simulink model "HDVSim_BEV_WPT_Truck"  (R2018b) (continued)
== system HDVSim_BEV_WPT_Truck/Electric == (continued)
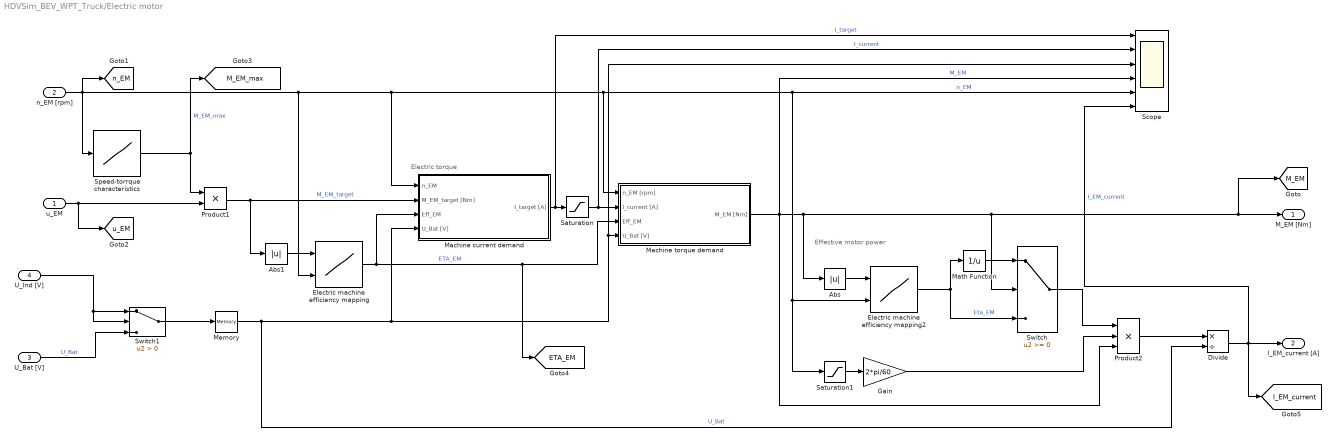
== system HDVSim_BEV_WPT_Truck/Electric motor ==
  note: Electric torque
  note: Effective motor power
  u_EM : Inport
  n_EM [rpm] : Inport  Port=2
  U_Bat [V] : Inport  Port=3
  U_Ind [V] : Inport  Port=4
  Abs : Abs
  Abs1 : Abs
  Divide : Product  Inputs=*/
  Electric machine efficiency mapping : Lookup_n-D  BreakpointsForDimension1=em.efficiency.torque  BreakpointsForDimension2=em.efficiency.speed  Table=em.efficiency.characteristic_map  RndMeth=Simplest  InputSameDT=on
  Electric machine efficiency mapping2 : Lookup_n-D  BreakpointsForDimension1=em.efficiency.torque  BreakpointsForDimension2=em. efficiency.speed  Table=em.efficiency.characteristic_map  RndMeth=Simplest  InputSameDT=on
  Gain : Gain  Gain=2*pi/60
  Machine current demand : Subsystem
  Machine torque demand : Subsystem
  Math Function : Math  Operator=reciprocal  SaturateOnIntegerOverflow=on
  Memory : Memory  InitialCondition=1.0943*Bat.Voltage
  Product1 : Product
  Product2 : Product  Inputs=3
  Saturation : Saturate  UpperLimit=600  LowerLimit=-600
  Saturation1 : Saturate  UpperLimit=inf  LowerLimit=1
  Scope : Scope  NumInputPorts=6
  Speed-torrque characteristics : Lookup_n-D  NumberOfTableDimensions=1  BreakpointsForDimension1=em.speed  Table=em.trq  ExtrapMethod=Clip  RndMeth=Simplest  InputSameDT=on
  Switch : Switch
  Switch1 : Switch  Criteria=u2 > Threshold
  M_EM [Nm] : Outport
  I_EM_current [A] : Outport  Port=2
  tag scope: ETA_EM=global, I_EM_current=global, M_EM=global, M_EM_max=global, n_EM=global, u_EM=global
  wires:
  Speed-torrque characteristics/1 -> [M_EM_max], Product1/1  "M_EM_max"
  u_EM/1 -> Product1/2, [u_EM]
  U_Bat [V]/1 -> Switch1/3  "U_Bat"
  Machine current demand/1(I_target [A]) -> Scope/1, Saturation/1  "I_target"
  Saturation/1 -> Scope/2, Machine torque demand/2(I_current [A])  "I_current"
  Machine torque demand/1(M_EM [Nm]) -> Scope/4, Product2/3, [M_EM], M_EM [Nm]/1, Switch/2, Abs/1  "M_EM"
  Gain/1 -> Product2/2
  Divide/1 -> [I_EM_current], Scope/6, I_EM_current [A]/1  "I_EM_current"
  Math Function/1 -> Switch/1
  Electric machine efficiency mapping2/1 -> Switch/3, Math Function/1  "Eta_EM"
  Switch/1 -> Product2/1
  Product2/1 -> Divide/1
  Abs/1 -> Electric machine efficiency mapping2/1
  Product1/1 -> Machine current demand/2(M_EM_target [Nm]), Abs1/1  "M_EM_target"
  n_EM [rpm]/1 -> Speed-torrque characteristics/1, Electric machine efficiency mapping/2, Machine current demand/1("n_EM "), Machine torque demand/1(n_EM [rpm]), Saturation1/1, Electric machine efficiency mapping2/2, Scope/5, [n_EM]  "n_EM"
  Abs1/1 -> Electric machine efficiency mapping/1
  Electric machine efficiency mapping/1 -> Machine current demand/3(Eff_EM), [ETA_EM], Machine torque demand/3(Eff_EM)  "ETA_EM"
  Saturation1/1 -> Gain/1
  Memory/1 -> Divide/2, Scope/3, Machine torque demand/4(U_Bat [V]), Machine current demand/4(U_Bat [V])  "U_Bat"
  Switch1/1 -> Memory/1
  U_Ind [V]/1 -> Switch1/2, Switch1/1

== system HDVSim_BEV_WPT_Truck/Hybrid ==
  Abs : Abs
  Abs1 : Abs
  Constant1 : Constant  Value=0
  Display1 : Display
  Display5 : Display
  Display6 : Display
  Gain1 : Gain  Gain=1/3600000
  Gain6 : Gain  Gain=1/3600000
  Gain7 : Gain  Gain=1/3600000
  Integrator1 : Integrator
  Integrator5 : Integrator
  Integrator6 : Integrator
  Out_Bat : Scope  NumInputPorts=4
  Product1 : Product
  Product3 : Product
  Scope1 : Scope
  Scope3 : Scope
  Scope7 : Scope  NumInputPorts=2
  Switch1 : Switch  Threshold=1
  wires:
  Product1/1 -> Switch1/1, Integrator6/1
  Gain1/1 -> Scope7/1, Display1/1  "E_REKUP_ES_AN [kWh]"
  Constant1/1 -> Switch1/3
  Integrator1/1 -> Gain1/1
  Switch1/1 -> Integrator1/1
  [REKUP] -> Scope7/2, Switch1/2  "REKUP"
  [U_Bat] -> Product1/2
  [I_current_BAT] -> Product1/1
  [SOC] -> Out_Bat/1
  Gain7/1 -> Display6/1, Scope1/1
  Integrator6/1 -> Gain7/1
  [U_Bat] -> Abs1/1
  [I_current_BAT] -> Abs/1
  Product3/1 -> Integrator5/1
  Gain6/1 -> Display5/1, Scope3/1
  Integrator5/1 -> Gain6/1
  Abs/1 -> Product3/1
  Abs1/1 -> Product3/2
  [s_current1] -> Out_Bat/2
  [C_Rate] -> Out_Bat/3
  [I_EM_current] -> Out_Bat/4

== system HDVSim_BEV_WPT_Truck/Inductive charging ==
  v_target [m/s] : Inport
  Compare To Constant1 : simulink/Logic and Bit Operations/Compare To Constant  relop=<=  const=WPT.expansion  OutDataTypeStr=boolean
  Compare To Constant2 : simulink/Logic and Bit Operations/Compare To Constant  relop=>=  const=78  OutDataTypeStr=boolean
  Compare To Constant3 : simulink/Logic and Bit Operations/Compare To Constant  relop=>  const=0  OutDataTypeStr=boolean
  Constant : Constant  Value=WPT.Voltage
  Constant1 : Constant  Value=0
  Gain : Gain  Gain=3.6
  Logical Operator : Logic  AllPortsSameDT=off  OutDataTypeStr=boolean
  Rate Transition : RateTransition
  Scope : Scope
  Scope1 : Scope  NumInputPorts=2
  Switch : Switch  Criteria=u2 ~= 0  Threshold=78
  Terminator : Terminator
  Uniform Random Number1 : UniformRandomNumber  Minimum=0  SampleTime=10
  U_Ind : Outport
  wires:
  v_target [m/s]/1 -> Gain/1
  Gain/1 -> Compare To Constant2/1
  Constant/1 -> Switch/1
  Constant1/1 -> Switch/3
  Switch/1 -> Scope/1, U_Ind/1
  Uniform Random Number1/1 -> Rate Transition/1
  Compare To Constant1/1 -> Logical Operator/1, Scope1/2
  Logical Operator/1 -> Switch/2
  Compare To Constant2/1 -> Logical Operator/2
  Rate Transition/1 -> Scope1/1, Compare To Constant1/1
  [slope_q] -> Compare To Constant3/1
  Compare To Constant3/1 -> Terminator/1

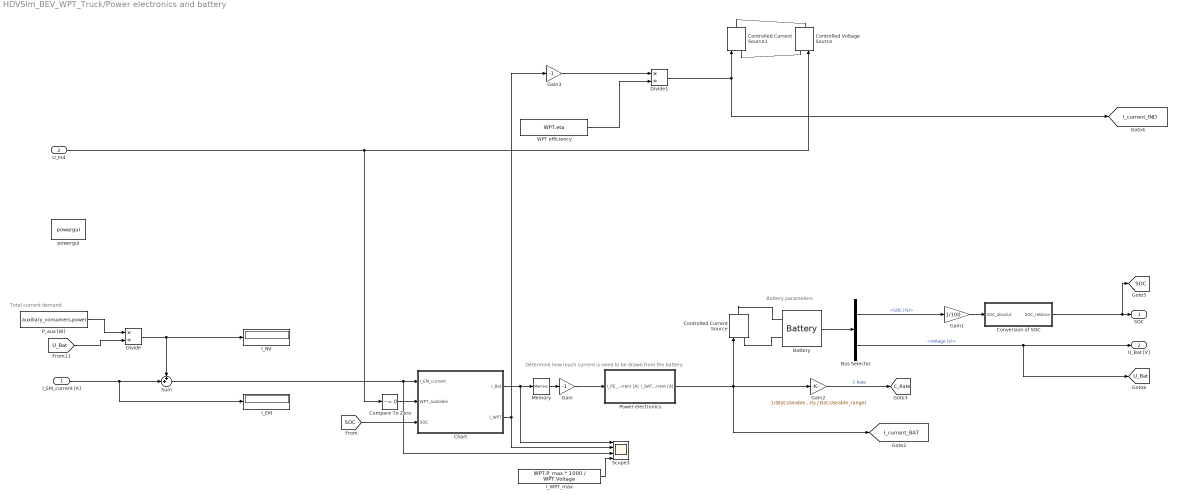
== system HDVSim_BEV_WPT_Truck/Power electronics and battery ==
  note: Total current demand
  note: Battery parameters
  note: Determine how much current is need to be drawn from the battery
  I_EM_current [A] : Inport
  U_Ind : Inport  Port=2
  Battery : electricdrivelib/Extra Sources/Battery  BatType=Lithium-Ion  ShowTempParam=off  ThermalPreset=no  ShowAgeParam=off  NomV=Bat.Voltage  NomQ=Bat.Useable_capacity / Bat.Useable_range  SOC=(Bat.SOC_start *Bat.Useable_range+(0.5-Bat.Useable_range/2))* 100  Batt_Tr=30  PresetModel=off  MaxQ=Bat.Useable_capacity / Bat.Useable_range *8077/7500  MinV=487.5  FullV=Bat.Voltage * 1.178  Dis_rate=0.2 * Bat.Useable_capacity / Bat.Useable_range  R=0.01 * Bat.Voltage / (Bat.Useable_capacity / Bat.Useable_range)  Normal_OP=Bat.Useable_capacity / Bat.Useable_range * 0.962  expZone="[Bat.Voltage*4339/4000     Bat.Useable_capacity / Bat.Useable_range/5]"  current=[5 25 50 250]  Units=Ampere-hour  Tci=20  NomT=20  T2=-30  MaxQ2=4.8  FullV2=7.1  Normal_OP2=5.72  expZone2="[6.58  1]"  Rca=0.6  ti=1000  Dp=0  Neq0=0  Tsim=1e6  Ta1=25  Qeol=5.4*0.9  Reol=0.013333*1.2  Char_age=[2.3478, 3]  Dis_age=[2.3478, 10]  N1=1500  N2=10500  N3=1000  N4=1400  Ta2=45  N5=950
      settings: SourceProductName=Simscape Electrical
  Bus Selector : BusSelector  OutputSignals=SOC (%),Voltage (V)
  Chart : Stateflow chart  TreatAsAtomicUnit=on
  Compare To Zero : simulink/Logic and Bit Operations/Compare To Zero  relop=~=  OutDataTypeStr=boolean
  Controlled Current Source : powerlib/Electrical Sources/Controlled Current Source  Initialize=off  Source_Type=DC  Amplitude=0  Phase=0  Frequency=0  Measurements=None
      settings: SourceProductName=Simscape Electrical
  Controlled Current Source1 : powerlib/Electrical Sources/Controlled Current Source  Initialize=off  Source_Type=DC  Amplitude=0  Phase=0  Frequency=0  Measurements=None
      settings: SourceProductName=Simscape Electrical
  Controlled Voltage Source : powerlib/Electrical Sources/Controlled Voltage Source  Initialize=on  Source_Type=DC  Amplitude=0  Phase=0  Frequency=0  Measurements=None
      settings: SourceProductName=Simscape Electrical
  Conversion of SOC : Subsystem
  Divide : Product  Inputs=*/
  Divide1 : Product  Inputs=*/
  Gain : Gain  Gain=-1
  Gain1 : Gain  Gain=1/100
  Gain2 : Gain  Gain=1/(Bat.Useable_capacity / Bat.Useable_range)
  Gain3 : Gain  Gain=-1
  I_EM : Display
  I_NV : Display
  I_WPT_max : Constant  Value=WPT.P_max * 1000 / WPT.Voltage
  Memory : Memory
  P_aux [W] : Constant  Value=auxiliary_consumers.power
  Power electronics : Subsystem
  Scope3 : Scope  NumInputPorts=4
  Sum : Sum  Inputs=++|
  WPT efficiency : Constant  Value=WPT.eta
  powergui : powerlib/powergui  Priority=1  SimulationMode=Discrete  SampleTime=sample_time  frequency=60  Iterations=50  frequencyindice=0  Pbase=100e6  ErrMax=1e-4  UnitsV=kV  UnitsW=MW  buscounter=0  FunctionMessages=off  echomessages=off  EnableUseOfTLC=off  CurrentSourceSwitches=off  DisableSnubberDevices=off  DisableRonSwitches=off  DisableVfSwitches=off  DisplayEquations=off  SolverType=Tustin  Interpol=off  ExternalGateDelay=off  methode=off  Ts=100  x0status=blocks  SPID=off  SwTol=0  HookPort=off  RestoreLinks=warning  ResistiveCurrentMeasurement=off  Frange=[0:2:500]  Ylog=off  Xlog=on  ShowGrid=off  save=off  variable=ZData  structure=0  ZoomFFT=off  StartTime=0.0  cycles=1  DisplayStyle=1  fundamental=60  FreqAxis=off  MaxFrequency=1000  frequencyindicesteady=1  RmsSteady=1  display=off
      settings: SourceProductName=Simscape Electrical
  SOC : Outport
  U_Bat [V] : Outport  Port=2
  tag scope: C_Rate=global, I_current_BAT=global, I_current_IND=global, SOC=global, U_Bat=global
  wires:
  Battery/1 -> Bus Selector/1
  Bus Selector/1 -> Gain1/1  "<SOC (%)>"
  Bus Selector/2 -> [U_Bat], U_Bat [V]/1  "<Voltage (V)>"
  Gain1/1 -> Conversion of SOC/1(SOC_absolut)
  Conversion of SOC/1(SOC_relative) -> [SOC], SOC/1
  Memory/1 -> Gain/1
  Gain/1 -> Power electronics/1(I_PE_current [A])
  Gain2/1 -> [C_Rate]  "C Rate"
  [U_Bat] -> Divide/2
  P_aux [W]/1 -> Divide/1
  Divide/1 -> Sum/1, I_NV/1
  Sum/1 -> Scope3/3, Chart/1(I_EM_current)
  U_Ind/1 -> Controlled Voltage Source/1, Compare To Zero/1
  Chart/1(I_Bat) -> Memory/1, Scope3/1
  Chart/2(I_WPT) -> Scope3/2, Gain3/1
  Compare To Zero/1 -> Chart/2(WPT_available)
  Gain3/1 -> Divide1/1
  Divide1/1 -> [I_current_IND], Controlled Current Source1/1
  WPT efficiency/1 -> Divide1/2
  I_WPT_max/1 -> Scope3/4
  Power electronics/1(I_BAT_current [A]) -> [I_current_BAT], Gain2/1, Controlled Current Source/1
  [SOC] -> Chart/3(SOC)
  I_EM_current [A]/1 -> I_EM/1, Sum/2
  connections:
  Controlled Current Source/LConn1 -- Battery/LConn2
  Controlled Current Source/RConn1 -- Battery/LConn1
  Controlled Current Source1/RConn1 -- Controlled Voltage Source/RConn1
  Controlled Current Source1/LConn1 -- Controlled Voltage Source/LConn1

== system HDVSim_BEV_WPT_Truck/Tires ==
  M_tire [Nm] : Inport
  v_current [m/s] : Inport  Port=2
  F=M/r : Product  Inputs=*/
  "phi. -> n[1/min]" : Gain  Gain=60/2/pi
  phi. = v/r : Product  Inputs=/*
  tire radius : Constant  Value=tires.radius
  n_tire [rpm] : Outport
  F_x [N] : Outport  Port=2
  wires:
  phi. = v/r/1 -> "phi. -> n[1/min]"/1
  "phi. -> n[1/min]"/1 -> n_tire [rpm]/1
  tire radius/1 -> phi. = v/r/1, F=M/r/2
  v_current [m/s]/1 -> phi. = v/r/2
  M_tire [Nm]/1 -> F=M/r/1
  F=M/r/1 -> F_x [N]/1

== system HDVSim_BEV_WPT_Truck/Transmission ==
  note: Transmission torque multiplication through the different gears
  M_EM [Nm] : Inport
  n_Gearbox [rpm] : Inport  Port=2
  delta_v : Inport  Port=3
  v_target [m/s] : Inport  Port=4
  alpha [rad] : Inport  Port=5
  Determine when shifting occurs : Subsystem  TreatAsAtomicUnit=on
  Memory1 : Memory
  Number of gears : Constant  Value=length(transmission.ratios)
  Saturation : Saturate  UpperLimit=inf  LowerLimit=0
  Shifting rules : Stateflow chart  TreatAsAtomicUnit=on
  Torque flow through the transmission : Subsystem
  ZKU : Subsystem
  init_gear : Constant  Value=transmission.init_gear
  n_EM [rpm] : Outport
  M_Gearbox [Nm] : Outport  Port=2
  tag scope: gear=global, i_gearbox=global, recommend_DOWN_now=global, recommend_UP_now=global
  wires:
  n_Gearbox [rpm]/1 -> Torque flow through the transmission/2(n_gearbox_out [1/min]), Determine when shifting occurs/2(n_Getr_out [1/min])
  Torque flow through the transmission/2(M_gearbox_out [Nm]) -> [M_Getr_out], M_Gearbox [Nm]/1
  Torque flow through the transmission/1(n_gearbox_in [1/min]) -> Saturation/1
  init_gear/1 -> Shifting rules/1(init_gear)
  Determine when shifting occurs/27(check_torque_reseve_2lower) -> Shifting rules/29(check_torque_reserve_2lower)
  Determine when shifting occurs/26(check_torque_reseve_2higher) -> Shifting rules/28(check_torque_reserve_2higher)
  Determine when shifting occurs/1(reccommend_down_2higher) -> Shifting rules/3(recommend_down_2higher)
  Determine when shifting occurs/2(reccommend_down_1higher) -> Shifting rules/4(recommend_down_1higher)
  Determine when shifting occurs/3(reccommend_down_now) -> Shifting rules/5(recommend_down_now), [recommend_DOWN_now]
  Determine when shifting occurs/4(reccommend_down_1lower) -> Shifting rules/6(recommend_down_1lower)
  Determine when shifting occurs/5(reccommend_down_2lower) -> Shifting rules/7(recommend_down_2llower)
  Determine when shifting occurs/6(reccommend_up_2higher) -> Shifting rules/8(recommend_up_2higher)
  Determine when shifting occurs/7(reccommend_up_1higher) -> Shifting rules/9(recommend_up_1higher)
  Determine when shifting occurs/8(reccommend_up_now) -> Shifting rules/10(recommend_up_now), [recommend_UP_now]
  Determine when shifting occurs/9(reccommend_up_1lower) -> Shifting rules/11(recommend_up_1lower)
  Determine when shifting occurs/10(reccommend_up_2lower) -> Shifting rules/12(recommend_up_2lower)
  Determine when shifting occurs/11(check_n_min_2higher) -> Shifting rules/13(check_n_min_2higher)
  Determine when shifting occurs/12(check_n_min_1higher) -> Shifting rules/14(check_n_min_1higher)
  Determine when shifting occurs/13(check_n_min_now) -> Shifting rules/15(check_n_min_now)
  Determine when shifting occurs/14(check_n_min_1lower) -> Shifting rules/16(check_n_min_1lower)
  Determine when shifting occurs/15(check_n_min_2lower) -> Shifting rules/17(check_n_min_2lower)
  Determine when shifting occurs/16(check_n_max_2higher) -> Shifting rules/18(check_n_max_2higher)
  Determine when shifting occurs/17(check_n_max_1higher) -> Shifting rules/19(check_n_max_1higher)
  Determine when shifting occurs/18(check_n_max_now) -> Shifting rules/20(check_n_max_now)
  Determine when shifting occurs/19(check_n_max_1lower) -> Shifting rules/21(check_n_max_1lower)
  Determine when shifting occurs/20(check_n_max_2lower) -> Shifting rules/22(check_n_max_2lower)
  Determine when shifting occurs/21(check_M_max_2higher) -> Shifting rules/23(check_M_max_2higher)  "check_M_max_2higher"
  Determine when shifting occurs/22(check_M_max_1higher) -> Shifting rules/24(check_M_max_1higher)  "check_M_max_1higher"
  Determine when shifting occurs/23(check_M_max_now) -> Shifting rules/25(check_M_max_now)  "check_M_max_now"
  Determine when shifting occurs/24(check_M_max_1lower) -> Shifting rules/26(check_M_max_1lower)  "check_M_max_1lower"
  Determine when shifting occurs/25(check_M_max_2lower) -> Shifting rules/27(check_M_max_2lower)  "check_M_max_2lower"
  Memory1/1 -> [gear], [Gear], ZKU/1(Gear), Torque flow through the transmission/3(Gear)
  M_EM [Nm]/1 -> Torque flow through the transmission/1(M_gearbox_in [Nm])
  Saturation/1 -> n_EM [rpm]/1
  Torque flow through the transmission/3(i_gearbox) -> [i_gearbox]
  delta_v/1 -> Determine when shifting occurs/4(delta_v)
  v_target [m/s]/1 -> Determine when shifting occurs/5(v_target [m/s])
  Number of gears/1 -> Shifting rules/2(z)
  alpha [rad]/1 -> Determine when shifting occurs/6(alpha [rad])
  Shifting rules/1(gear) -> [gear]
  [gear] -> Memory1/1
  [M_Getr_out] -> Determine when shifting occurs/3(M_Getr_out [Nm])
  [Gear] -> Determine when shifting occurs/1(Gear)

== system HDVSim_BEV_WPT_Truck/Vehicle ==
  note: Net tractive force applied on the vehicle
  note: Vehicle acceleration
  F_x [N] : Inport
  u_KB : Inport  Port=2
  alpha [rad] : Inport  Port=3
  Add : Sum  Inputs=-+-
  Braking force [N] : Product  RndMeth=Zero
  Cargo weight : Constant  Value=vehicle.payload
  Driving resistances without acceleration : Subsystem
  F_brake_max : Constant  Value=vehicle.F_brake_max
  Integrator : Integrator
  Integrator1 : Integrator  InitialCondition=cycle.speed_init  LimitOutput=on  LowerSaturationLimit=0
  Product : Product  Inputs=3
  Product2 : Product  Inputs=*/  RndMeth=Zero
  Product3 : Product  Inputs=**  RndMeth=Zero
  Sum1 : Sum  Inputs=|++
  Sum2 : Sum  Inputs=|++
  Vehicle weight : Constant  Value=vehicle.mass
  v_current [m/s] : Outport
  s_current [m] : Outport  Port=2
  tag scope: acceleration_drag=global, s_current1=global, v_current1=global
  wires:
  alpha [rad]/1 -> Driving resistances without acceleration/1(alpha [rad])
  Add/1 -> Product2/1
  Driving resistances without acceleration/1(F_W [N]) -> Add/3
  F_x [N]/1 -> Add/2  "F_x"
  F_brake_max/1 -> Braking force [N]/2
  Integrator/1 -> [s_current1], s_current [m]/1  "s_current"
  Cargo weight/1 -> Sum1/2, Sum2/2
  Sum1/1 -> Product2/2
  Vehicle weight/1 -> Sum2/1, Product3/2
  Product3/1 -> Sum1/1
  Integrator1/1 -> v_current [m/s]/1, Driving resistances without acceleration/2(v [m/s]), [v_current1], Integrator/1  "v_current"
  [lamda] -> Product/1, Product3/1
  u_KB/1 -> Braking force [N]/1
  Braking force [N]/1 -> Add/1  "F_Brake"
  Product2/1 -> Integrator1/1, Product/3  "a"
  Product/1 -> [acceleration_drag]
  Sum2/1 -> Product/2

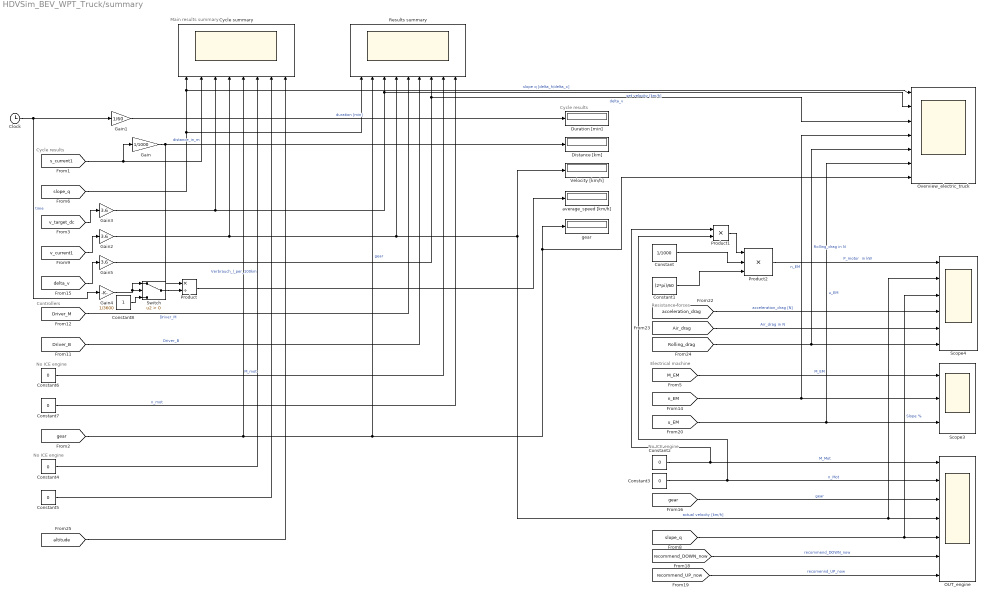
== system HDVSim_BEV_WPT_Truck/summary ==
  note: Cycle results
  note: Controllers
  note: No ICE engine
  note: No ICE engine
  note: Main results summary
  note: Cycle results
  note: Resistance forces
  note: Electrical machine
  note: No ICE engine
  Clock : Clock
  Constant : Constant  Value=1/1000
  Constant1 : Constant  Value=(2*pi)/60
  Constant{2..7} (6 alike) : Constant  Value=0
  Constant8 : Constant
  Cycle summary : Scope  NumInputPorts=8
  Distance [km] : Display
  Duration [min] : Display
  Gain : Gain  Gain=1/1000
  Gain1 : Gain  Gain=1/60
  Gain2 : Gain  Gain=3.6
  Gain3 : Gain  Gain=3.6
  Gain4 : Gain  Gain=1/3600
  Gain5 : Gain  Gain=3.6
  OUT_engine : Scope  NumInputPorts=7
  Overview_electric_truck : Scope  NumInputPorts=7
  Product : Product  Inputs=*/  RndMeth=Zero
  Product1 : Product
  Product2 : Product  Inputs=3
  Results summary : Scope  NumInputPorts=9
  Scope3 : Scope  NumInputPorts=3
  Scope4 : Scope  NumInputPorts=6
  Switch : Switch  Criteria=u2 > Threshold
  Velocity [km/h] : Display
  average_speed [km/h] : Display
  gear : Display
  wires:
  [v_current1] -> Gain2/1
  [s_current1] -> Cycle summary/2, Gain/1  "distance_in_m"
  Gain/1 -> Distance [km]/1, Product/1
  Clock/1 -> Gain1/1, Gain4/1  "time"
  Gain1/1 -> Duration [min]/1  "duration [min]"
  Gain2/1 -> Cycle summary/4, OUT_engine/4, Scope4/2, Velocity [km/h]/1, Results summary/4  "actual velocity [km/h]"
  [gear] -> gear/1, Overview_electric_truck/7, Results summary/2, Cycle summary/5  "gear"
  [v_target_dc] -> Gain3/1
  [slope_q] -> Results summary/1, Overview_electric_truck/1, Cycle summary/1  "slope q [delta_h/delta_x]"
  Constant4/1 -> Cycle summary/6  "Verbrauch_l_per_100km"
  Gain3/1 -> Results summary/3, Overview_electric_truck/2, Cycle summary/3  "set velocity [km/h]"
  Gain4/1 -> Switch/1, Switch/2
  Product/1 -> average_speed [km/h]/1
  Constant5/1 -> Cycle summary/7
  [Driver_M] -> Results summary/5  "Driver_M"
  [Driver_B] -> Results summary/6  "Driver_B"
  Constant6/1 -> Results summary/8  "M_mot"
  [delta_v] -> Gain5/1
  Constant2/1 -> OUT_engine/1, Product1/1  "M_Mot"
  Constant3/1 -> Product1/2, OUT_engine/2  "n_Mot"
  [gear] -> OUT_engine/3  "gear"
  [recommend_DOWN_now] -> OUT_engine/6  "recommend_DOWN_now"
  [recommend_UP_now] -> OUT_engine/7  "recomennd_UP_now"
  [slope_q] -> Scope4/3, OUT_engine/5  "Slope %"
  Gain5/1 -> Results summary/7, Overview_electric_truck/3  "delta_v"
  Constant7/1 -> Results summary/9  "n_mot"
  [M_EM] -> Scope3/1  "M_EM"
  [n_EM] -> Overview_electric_truck/4, Scope3/2  "n_EM"
  [u_EM] -> Overview_electric_truck/6, Scope3/3  "u_EM"
  Product1/1 -> Product2/1
  Constant/1 -> Product2/2
  Product2/1 -> Scope4/1  "P_motor  in kW"
  Constant1/1 -> Product2/3
  [acceleration_drag] -> Scope4/4  "acceleration_drag [N]"
  [Air_drag] -> Scope4/5  "Air_drag in N"
  [Rolling_drag] -> Overview_electric_truck/5, Scope4/6  "Rolling_drag in N"
  Switch/1 -> Product/2
  Constant8/1 -> Switch/3
  [altitude] -> Cycle summary/8

== system HDVSim_BEV_WPT_Truck/Active cruise control/Braking from upper speed limit ==
  v_target [m/s] : Inport
  Add3 : Sum  Inputs=+-
  Constant1 : Constant  Value=90/3.6
  Constant10 : Constant  Value=prospective_cruise_control
  Constant6 : Constant  Value=v_PPC_delta
  Constant7 : Constant  Value=0
  Constant8 : Constant  Value=0
  Saturation Dynamic : simulink/Discontinuities/Saturation Dynamic  OutMin=[]  OutMax=[]  OutDataTypeStr=Inherit: Same as second input  OutDataType=fixdt(1, 16)  OutScaling=2^-10  DoSatur=off
  Switch3 : Switch  Criteria=u2 > Threshold
  Out1 : Outport
  wires:
  Switch3/1 -> Out1/1
  Constant6/1 -> Saturation Dynamic/2
  Constant1/1 -> Add3/1
  Constant7/1 -> Switch3/3
  Add3/1 -> Saturation Dynamic/1
  Constant8/1 -> Saturation Dynamic/3
  Constant10/1 -> Switch3/2
  v_target [m/s]/1 -> Add3/2
  Saturation Dynamic/1 -> Switch3/1

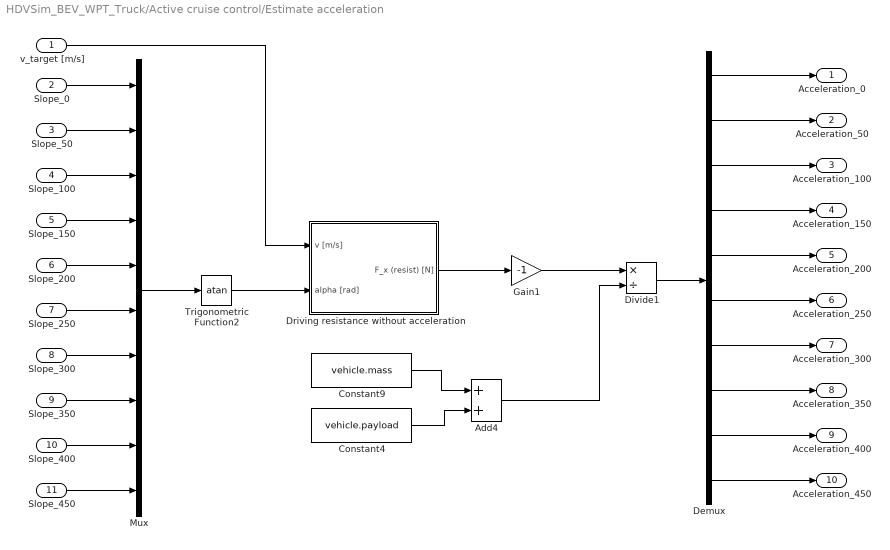
== system HDVSim_BEV_WPT_Truck/Active cruise control/Estimate acceleration ==
  v_target [m/s] : Inport
  Slope_0 : Inport  Port=2
  Slope_50 : Inport  Port=3
  Slope_100 : Inport  Port=4
  Slope_150 : Inport  Port=5
  Slope_200 : Inport  Port=6
  "Slope_250 " : Inport  Port=7
  Slope_300 : Inport  Port=8
  Slope_350 : Inport  Port=9
  Slope_400 : Inport  Port=10
  Slope_450 : Inport  Port=11
  Add4 : Sum
  Constant4 : Constant  Value=vehicle.payload
  Constant9 : Constant  Value=vehicle.mass
  Demux : Demux  Outputs=10
  Divide1 : Product  Inputs=*/
  Driving resistance without acceleration : Subsystem
  Gain1 : Gain  Gain=-1
  Mux : Mux  Inputs=10
  Trigonometric Function2 : Trigonometry  Operator=atan
  Acceleration_0 : Outport
  Acceleration_50 : Outport  Port=2
  Acceleration_100 : Outport  Port=3
  Acceleration_150 : Outport  Port=4
  Acceleration_200 : Outport  Port=5
  Acceleration_250 : Outport  Port=6
  Acceleration_300 : Outport  Port=7
  Acceleration_350 : Outport  Port=8
  Acceleration_400 : Outport  Port=9
  Acceleration_450 : Outport  Port=10
  wires:
  v_target [m/s]/1 -> Driving resistance without acceleration/1(v [m/s])
  Trigonometric Function2/1 -> Driving resistance without acceleration/2(alpha [rad])
  Constant9/1 -> Add4/1
  Constant4/1 -> Add4/2
  Driving resistance without acceleration/1(F_x (resist) [N]) -> Gain1/1
  Add4/1 -> Divide1/2
  Gain1/1 -> Divide1/1
  Mux/1 -> Trigonometric Function2/1
  Divide1/1 -> Demux/1
  Demux/1 -> Acceleration_0/1
  Slope_0/1 -> Mux/1
  Slope_50/1 -> Mux/2
  Slope_100/1 -> Mux/3
  Slope_150/1 -> Mux/4
  Slope_200/1 -> Mux/5
  "Slope_250 "/1 -> Mux/6
  Slope_300/1 -> Mux/7
  Slope_350/1 -> Mux/8
  Slope_400/1 -> Mux/9
  Slope_450/1 -> Mux/10
  Demux/2 -> Acceleration_50/1
  Demux/3 -> Acceleration_100/1
  Demux/4 -> Acceleration_150/1
  Demux/5 -> Acceleration_200/1
  Demux/6 -> Acceleration_250/1
  Demux/7 -> Acceleration_300/1
  Demux/8 -> Acceleration_350/1
  Demux/9 -> Acceleration_400/1
  Demux/10 -> Acceleration_450/1

== system HDVSim_BEV_WPT_Truck/Active cruise control/Estimate critical slope ==
  note: Total resistive forces on the vehicle
  note: Friction resistance
  note: Drag on the vehicle
  note: Forward force applied on the vehicle
  v_target [m/s] : Inport
  A : Constant  Value=vehicle.frontal_area
  Add : Sum
  Add1 : Sum
  Add3 : Sum
  Constant1 : Constant  Value=vehicle.payload
  Constant4 : Constant  Value=addition_for_critical_slope
  Constant5 : Constant  Value=tires.roll_drag_coeff.total
  Constant8 : Constant  Value=vehicle.mass
  Differential efficiency : Constant  Value=final_drive.trq_eff
  Differential gear ratio : Constant  Value=final_drive.ratio
  Divide : Product  Inputs=***/**
  Divide1 : Product  Inputs=*/
  Product : Product
  Product2 : Product  Inputs=4  RndMeth=Zero
  Subtract : Sum  Inputs=+-
  Sum3 : Sum  Inputs=++|
  Tire radius : Constant  Value=tires.radius
  Trigonometric Function : Trigonometry  Operator=asin
  Trigonometric Function1 : Trigonometry  Operator=tan
  c_d : Constant  Value=vehicle.air_drag_coeff(end)
  g : Gain  Gain=ambient.gravity
  gain2 : Gain  Gain=0.5
  rho : Constant  Value=ambient.air_density
  v² : Math  Operator=square  SaturateOnIntegerOverflow=on
  critical_slope : Outport
  wires:
  Add3/1 -> Divide/3
  [i_gearbox] -> Divide/1
  Differential gear ratio/1 -> Divide/2
  Tire radius/1 -> Divide/4
  Divide/1 -> Subtract/1
  Subtract/1 -> Divide1/1  "F_net"
  v²/1 -> Product2/1
  rho/1 -> Product2/2
  A/1 -> Product2/3
  c_d/1 -> Product2/4
  Product2/1 -> gain2/1
  Constant8/1 -> Add1/1
  Constant1/1 -> Add1/2
  Add1/1 -> g/1
  Constant5/1 -> Product/2
  Trigonometric Function/1 -> Trigonometric Function1/1
  Divide1/1 -> Trigonometric Function/1
  Sum3/1 -> critical_slope/1
  Constant4/1 -> Sum3/1
  Trigonometric Function1/1 -> Sum3/2
  v_target [m/s]/1 -> v²/1
  [Eta_Transmission] -> Divide/5
  Differential efficiency/1 -> Divide/6
  [M_EM_max] -> Add3/1
  [M_EM_max] -> Add3/2
  gain2/1 -> Add/1
  Product/1 -> Add/2
  Add/1 -> Subtract/2  "F_resistive"
  g/1 -> Product/1, Divide1/2

== system HDVSim_BEV_WPT_Truck/Active cruise control_old/Braking from upper speed limit ==
  v_soll [m/s] : Inport
  Add3 : Sum  Inputs=+-
  Constant1 : Constant  Value=90/3.6
  Constant10 : Constant  Value=Vorausschauender_Tempomat
  Constant6 : Constant  Value=6/3.6
  Constant7 : Constant  Value=0
  Constant8 : Constant  Value=0
  Saturation Dynamic : simulink/Discontinuities/Saturation Dynamic  OutMin=[]  OutMax=[]  OutDataTypeStr=Inherit: Same as second input  OutDataType=fixdt(1, 16)  OutScaling=2^-10  DoSatur=off
  Switch3 : Switch  Criteria=u2 > Threshold
  Out1 : Outport
  wires:
  Switch3/1 -> Out1/1
  Constant6/1 -> Saturation Dynamic/2
  Constant1/1 -> Add3/1
  Constant7/1 -> Switch3/3
  Add3/1 -> Saturation Dynamic/1
  Constant8/1 -> Saturation Dynamic/3
  Constant10/1 -> Switch3/2
  v_soll [m/s]/1 -> Add3/2
  Saturation Dynamic/1 -> Switch3/1
